simulink model "ofdm_txrx_mimo"  (Simulink 7.1)
settings: Solver=ode45  StartTime=0.0  StopTime=6e3  RelTol=1e-3
PreLoadFcn:
  | ofdm_rx_mimo_init
InitFcn:
  | ofdm_rx_mimo_init
StartFcn:
  | ofdm_rx_mimo_init
values from neighbouring files (symbols this model uses):
  BER_RAM_init_size = 2048    [ofdm_tx_mimo_init.m]
  CPLength = 16    [ofdm_tx_mimo_init.m]
  CRCPolynomial = hex2dec('04c11db7')    [ofdm_tx_mimo_init.m]
  freqOffset_track_filtCoef_i = hex2dec('2500')/2^32    [ofdm_rx_mimo_init.m]
  freqOffset_track_filtCoef_p = hex2dec('d0000')/2^32    [ofdm_rx_mimo_init.m]
  hi = zeros(1,length(cci))    [ofdm_rx_mimo_init.m]
  hr = zeros(1,length(ccr))    [ofdm_rx_mimo_init.m]
  max_CP_length = 16    [ofdm_tx_mimo_init.m]
  max_num_baseRateSymbols = 31    [ofdm_tx_mimo_init.m]
  max_num_subcarriers = 64    [ofdm_tx_mimo_init.m]
  max_num_trainingSymbols = 15    [ofdm_tx_mimo_init.m]
  max_numBytes = 16384    [ofdm_tx_mimo_init.m]
  max_OFDM_symbols = 2047    [ofdm_tx_mimo_init.m]
  modConstellation_qam16 = .75*[1 3 -1 -3]./3    [ofdm_tx_mimo_init.m]
  modConstellation_qam256 = 0.9375*[3 1 5 7 11 9 13 15 -3 -1 -5 -7 -11 -9 -13 -15]./15    [ofdm_tx_mimo_init.m]
  modConstellation_qam64 = 0.875*[3 1 5 7 -3 -1 -5 -7]./7    [ofdm_tx_mimo_init.m]
  modConstellation_qpsk = [1 -1]./sqrt(2)    [ofdm_tx_mimo_init.m]
  numBaseRateSymbols = 2    [ofdm_tx_mimo_init.m]
  numSubcarriers = 64    [ofdm_tx_mimo_init.m]
  numTrainingSymbols = 2    [ofdm_tx_mimo_init.m]
  pilot1_indicies = 7 + ( (64-7) * 2^16)    (= 3735559)    [ofdm_tx_mimo_init.m]
  pilot2_indicies = 21 + ( (64-21) * 2^16)    (= 2818069)    [ofdm_tx_mimo_init.m]
  pilotValue_neg = hex2dec('8000') + (2^16 * 0)    [ofdm_tx_mimo_init.m]
  pilotValue_pos = hex2dec('7FFF') + (2^16 * 0)    [ofdm_tx_mimo_init.m]
  pkt_crossCorr_thresh = 0.7    [ofdm_rx_mimo_init.m]
  pkt_energy_thresh = 0    [ofdm_rx_mimo_init.m]
  pkt_numPayloadBytes = 1400+42-6    (= 1436)    [ofdm_tx_mimo_init.m]
  pktByteNums = numHeaderBytes +  				byteIndex_numPayloadBytes(1)*2^8 +  				byteIndex_numPayloadBytes(2)*2^16 +  				byteIndex_simpleDynModMasks * 2^24    [ofdm_rx_mimo_init.m]
  PktDet_Delay = 26 + Symbol_Timing_Offset*2^7    (= 1050)    [ofdm_rx_mimo_init.m]
  preamble = 6*[0 shortsyms_10 longsyms_2]    [ofdm_tx_mimo_init.m]
  preamble_I = real(preamble)    [ofdm_tx_mimo_init.m]
  preamble_Q = imag(preamble)    [ofdm_tx_mimo_init.m]
  RAM_init_size = max_numBytes    (= 16384)    [ofdm_tx_mimo_init.m]
  reg_Interrupt_PktBuf_Control = reg_InterruptControl +  	1 * 2^16 +  	0 * 2^24    (= 65656)    [ofdm_rx_mimo_init.m]
  rx_controlBits = 1 * 2  		 + 1 * 4  		 + 0 * 8  		 + 1 * 16 * rx_SISO_Mode  		 + 1 * 32  		 + 1 * 64  		 + 0 * 128  		 + 1 * 256  		 + 0 * 512  		 + 1 * 1024  		 + 0 * 2048  		 + 1 * 4096  		 + 1 * 8192  		 + 0 * 16384  		 + 1 * 65536  		 + 1 * 131072  		 + 0 * 262144  		 + 1 * 524288    [ofdm_rx_mimo_init.m]
  Rx_PhaseNoiseTrack_K = 0.6094    [ofdm_rx_mimo_init.m]
  rx_postEq_scaling = round(rxScaling*2^11) * (1 + 2^16)    [ofdm_rx_mimo_init.m]
  RxDataScrambling_Seq = [40 198 78 63 82 173 102 245 48 111 172 115 147 230 216 93 72 65 62 2 205 242 122 90 128 83 105 97 73 10 5 252]    [ofdm_tx_mimo_init.m]
  simOnly_numSamples = length(preamble)+( (numSubcarriers+CPLength)*(numTrainingSymbols + numBaseRateSymbols + numFullRateSymbols/2) )    [ofdm_tx_mimo_init.m]
  train = [train train]    [ofdm_tx_mimo_init.m]
  tx_controlBits = (antB_preambleShift * 2^4) + (2^0 * tx_SISO_Mode) + 4*0 + 8*1    (= 73)    [ofdm_tx_mimo_init.m]
  TxDataScrambling_Seq = [40 198 78 63 82 173 102 245 48 111 172 115 147 230 216 93 72 65 62 2 205 242 122 90 128 83 105 97 73 10 5 252]    [ofdm_tx_mimo_init.m]
  txReg_symbolCounts = (2^16 * numFullRateSymbols) + (2^8 *numBaseRateSymbols ) + numTrainingSymbols    [ofdm_tx_mimo_init.m]
  TxRx_FFTScaling = bin2dec('010111') + (bin2dec('000101') * 2^6)    [ofdm_tx_mimo_init.m]
undefined symbols (not defined in the file or in its folder): AntA, AntB, BaseRate, CRC_table_gen, gcbh, intfilt, pktTiming_count_payloadStart, pktTiming_symbol_length, xlBlockLoadCallback, xlGetSimulinkPeriod
libraries: xbsIndex_r4 x4212, dspmlti4 x4, dspsigops x4, commchan3 x2, simulink x2, commrflib2 x2, dspsrcs4 x1
(part 11 of 43)

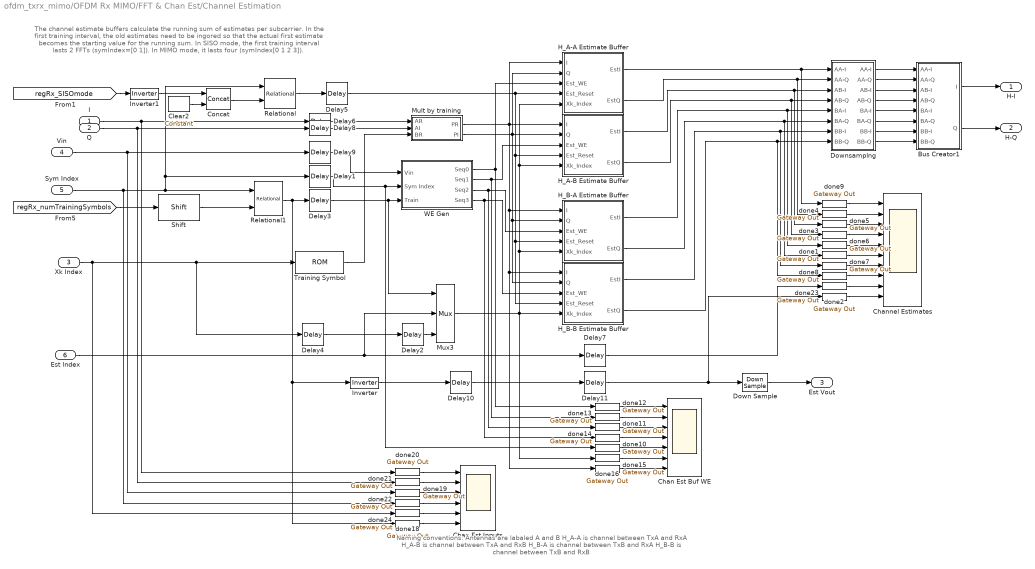
== system ofdm_txrx_mimo/OFDM Rx MIMO/FFT & Chan Est/Channel Estimation ==
  note: The channel estimate buffers calculate the
      | running sum of estimates per subcarrier. In the first
      | training interval, the old estimates need to be ingored
      | so that the actual first estimate becomes the starting value
      | for the running sum. In SISO mode, the first training
      | interval lasts 2 FFTs (symIndex=[0 1]). In MIMO
      | mode, it lasts four (symIndex[0 1 2 3]).
  note: Naming conventions:
      | Antennas are labaled A and B
      | H_A-A is channel between TxA and RxA
      | H_A-B is channel between TxA and RxB
      | H_B-A is channel between TxB and RxA
      | H_B-B is channel between TxB and RxB
  I : Inport  OutDataType=sfix(16)  OutScaling=2^0
  Q : Inport  Port=2  OutDataType=sfix(16)  OutScaling=2^0
  Xk Index : Inport  Port=3  OutDataType=sfix(16)  OutScaling=2^0
  Vin : Inport  Port=4  OutDataType=sfix(16)  OutScaling=2^0
  Sym Index : Inport  Port=5  OutDataType=sfix(16)  OutScaling=2^0
  Est Index : Inport  Port=6  OutDataType=sfix(16)  OutScaling=2^0
  Bus Creator1 : Subsystem
  Chan Est Buf WE : Scope  NumInputPorts=7
      settings: TimeRange=2000  YMin=0~0~0~0~0~0~-0.4  YMax=1~1~1~1~30~70~0.4  SaveName=ScopeData21
  Chan Est Inputs : Scope  NumInputPorts=6
      settings: TimeRange=1800  YMin=-0.01~-0.01~0~0~0~0  YMax=0.01~0.01~1~10~80~1  SaveName=ScopeData23
  Channel Estimates : Scope  NumInputPorts=10
      settings: TimeRange=3000  YMin=-0.2~-0.2~-1~-1~-1~-1~-1~-1~0~0.95  YMax=0.2~0.2~1~1~1~1~1~1~75~1.05  SaveName=ScopeData24
  Clear2 : xbsIndex_r4/Constant  arith_type=Unsigned  const=1  n_bits=1  bin_pt=0  explicit_period=off  period=1  equ=P=C  opselect=C  inp2=PCIN>>17  opr=+  inp1=P  carry=CIN  dbl_ovrd=off  block_type=constant
      settings: block_version=VER_STRING_GOES_HERE
  Concat : xbsIndex_r4/Concat  num_inputs=2  dbl_ovrd=off  block_type=concat
      settings: block_version=8.2.01
  Delay1 : xbsIndex_r4/Delay  en=off  latency=1  dbl_ovrd=off  reg_retiming=on  block_type=delay
      settings: infoedit=Hardware notes: A delay line is a chain, each link of which is an SRL16 followed by a flip-flop. If register retiming is enabled, the delay line is a chain of flip-flops.  xl_area=[0, 0, 0, 0, 0, 0, 0]  block_version=VER_STRING_GOES_HERE
  Delay{2,4..11} (9 alike) : xbsIndex_r4/Delay  en=off  latency=1  dbl_ovrd=off  reg_retiming=off  block_type=delay
      settings: infoedit=Hardware notes: A delay line is a chain, each link of which is an SRL16 followed by a flip-flop. If register retiming is enabled, the delay line is a chain of flip-flops.  xl_area=[0, 0, 0, 0, 0, 0, 0]  block_version=VER_STRING_GOES_HERE
  Delay3 : xbsIndex_r4/Delay  en=off  latency=1  dbl_ovrd=off  reg_retiming=on  block_type=delay
      settings: infoedit=Hardware notes: A delay line is a chain, each link of which is an SRL16 followed by a flip-flop. If register retiming is enabled, the delay line is a chain of flip-flops.  xl_area=[0, 0, 0, 0, 0, 0, 0]  block_version=VER_STRING_GOES_HERE
  Down Sample : xbsIndex_r4/Down Sample  sample_ratio=2  sample_phase="Last Value of Frame  (most efficient)"  en=off  latency=1  dbl_ovrd=off  block_type=dsamp
      settings: block_version=10.1.2
  Downsamplng : Subsystem
  H_A-A Estimate Buffer : Subsystem
  H_A-B Estimate Buffer : Subsystem
  H_B-A Estimate Buffer : Subsystem
  H_B-B Estimate Buffer : Subsystem
  Inverter : xbsIndex_r4/Inverter  en=off  latency=1  dbl_ovrd=off  block_type=inv
      settings: block_version=VER_STRING_GOES_HERE
  Inverter1 : xbsIndex_r4/Inverter  en=off  latency=0  dbl_ovrd=off  block_type=inv
      settings: block_version=VER_STRING_GOES_HERE
  Mult by training : Subsystem
  Mux3 : xbsIndex_r4/Mux  inputs=2  en=off  latency=0  precision=Full  arith_type="Signed  (2's comp)"  n_bits=8  bin_pt=2  quantization=Truncate  overflow=Wrap  dbl_ovrd=off  block_type=mux
      settings: xl_area=[0, 0, 0, 0, 0, 0, 0]  block_version=VER_STRING_GOES_HERE
  Relational : xbsIndex_r4/Relational  mode=a<=b  en=off  latency=0  dbl_ovrd=off  block_type=relational
      settings: xl_area=[0, 0, 0, 0, 0, 0, 0]  block_version=VER_STRING_GOES_HERE
  Relational1 : xbsIndex_r4/Relational  mode=a<b  en=off  latency=0  dbl_ovrd=off  block_type=relational
      settings: xl_area=[3 0 0 6 0 0 0]  block_version=VER_STRING_GOES_HERE
  Shift : xbsIndex_r4/Shift  shift_dir=Left  shift_bits=1  en=off  latency=0  precision=User Defined  arith_type=Unsigned  n_bits=1+ceil(log2(max_num_trainingSymbols))  bin_pt=0  quantization=Truncate  overflow=Flag as error  dbl_ovrd=off  block_type=shift
      settings: block_version=VER_STRING_GOES_HERE
  Training Symbol : xbsIndex_r4/ROM  depth=length(train)/2  initVector=sign(train)  distributed_mem=Block RAM  rst=off  init_reg=0  en=off  latency=1  arith_type="Signed  (2's comp)"  n_bits=2  bin_pt=1  dbl_ovrd=off  optimize=Area  use_rpm=off  block_type=sprom
      settings: xl_area=[0, 0, 0, 0, 0, 0, 0]  block_version=VER_STRING_GOES_HERE
  WE Gen : Subsystem
  done{1..16,18..24} (23 alike) : xbsIndex_r4/Gateway Out  hdl_port=off  timing_constraint=None  locs_specified=off  LOCs={}  block_type=gatewayout
      settings: infoedit="Gateway out block.  Converts Xilinx fixed point inputs into ouputs of type Simulink integer, double, or fixed point.<P><P>Hardware notes:  In hardware these blocks become top level output ports or are discarded, depending on how they are configured."  xl_area=[0 0 0 0 1 0 0]  block_version=VER_STRING_GOES_HERE
  H-I : Outport  OutDataType=sfix(16)  OutScaling=2^0
  H-Q : Outport  Port=2  OutDataType=sfix(16)  OutScaling=2^0
  Est Vout : Outport  Port=3  OutDataType=sfix(16)  OutScaling=2^0
  wires:
  Inverter1/1 -> Concat/1
  Clear2/1 -> Concat/2
  [regRx_SISOmode] -> Inverter1/1
  Delay5/1 -> H_B-B Estimate Buffer/4(Est_Reset), H_B-A Estimate Buffer/4(Est_Reset), H_A-B Estimate Buffer/4(Est_Reset), H_A-A Estimate Buffer/4(Est_Reset)
  Delay2/1 -> Mux3/3
  Est Index/1 -> Mux3/2, Delay7/1
  Delay10/1 -> Delay11/1
  done23/1 -> Channel Estimates/10
  done9/1 -> Channel Estimates/1
  done2/1 -> Channel Estimates/9
  done8/1 -> Channel Estimates/8
  done7/1 -> Channel Estimates/7
  done6/1 -> Channel Estimates/5
  done3/1 -> Channel Estimates/4
  done5/1 -> Channel Estimates/3
  done4/1 -> Channel Estimates/2
  done1/1 -> Channel Estimates/6
  H_B-B Estimate Buffer/2(EstQ) -> done8/1, Downsamplng/8(BB-Q)
  H_B-B Estimate Buffer/1(EstI) -> done7/1, Downsamplng/7(BB-I)
  H_B-A Estimate Buffer/2(EstQ) -> done1/1, Downsamplng/6(BA-Q)
  H_B-A Estimate Buffer/1(EstI) -> done6/1, Downsamplng/5(BA-I)
  H_A-B Estimate Buffer/2(EstQ) -> done3/1, Downsamplng/4(AB-Q)
  H_A-B Estimate Buffer/1(EstI) -> done5/1, Downsamplng/3(AB-I)
  H_A-A Estimate Buffer/2(EstQ) -> done4/1, Downsamplng/2(AA-Q)
  H_A-A Estimate Buffer/1(EstI) -> done9/1, Downsamplng/1(AA-I)
  Delay11/1 -> done23/1, Down Sample/1
  Inverter/1 -> Delay10/1
  Delay7/1 -> done2/1
  done24/1 -> Chan Est Inputs/6
  done22/1 -> Chan Est Inputs/4
  done19/1 -> Chan Est Inputs/3
  done21/1 -> Chan Est Inputs/2
  done20/1 -> Chan Est Inputs/1
  done18/1 -> Chan Est Inputs/5
  Delay9/1 -> WE Gen/1(Vin)
  Vin/1 -> done19/1, Delay9/1
  Delay8/1 -> Mult by training/2(AI)
  Delay6/1 -> Mult by training/1(AR)
  Delay3/1 -> Mux3/1, WE Gen/3(Train)
  Delay1/1 -> done10/1, WE Gen/2(Sym Index)
  [regRx_numTrainingSymbols] -> Shift/1
  done15/1 -> Chan Est Buf WE/7
  done16/1 -> Chan Est Buf WE/6
  done14/1 -> Chan Est Buf WE/4
  done11/1 -> Chan Est Buf WE/3
  done13/1 -> Chan Est Buf WE/2
  done12/1 -> Chan Est Buf WE/1
  done10/1 -> Chan Est Buf WE/5
  Mult by training/2(PI) -> H_B-B Estimate Buffer/2(Q), H_B-A Estimate Buffer/2(Q), H_A-A Estimate Buffer/2(Q), H_A-B Estimate Buffer/2(Q)
  Relational1/1 -> Inverter/1, done24/1, Delay3/1
  WE Gen/2(Seq1) -> done13/1, H_A-B Estimate Buffer/3(Est_WE)
  WE Gen/4(Seq3) -> done14/1, H_B-B Estimate Buffer/3(Est_WE)
  WE Gen/3(Seq2) -> done11/1, H_B-A Estimate Buffer/3(Est_WE)
  WE Gen/1(Seq0) -> done12/1, H_A-A Estimate Buffer/3(Est_WE)
  Mux3/1 -> done16/1, H_A-A Estimate Buffer/5(Xk_Index), H_A-B Estimate Buffer/5(Xk_Index), H_B-A Estimate Buffer/5(Xk_Index), H_B-B Estimate Buffer/5(Xk_Index)
  Mult by training/1(PR) -> done15/1, H_B-B Estimate Buffer/1(I), H_B-A Estimate Buffer/1(I), H_A-B Estimate Buffer/1(I), H_A-A Estimate Buffer/1(I)
  Relational/1 -> Delay5/1
  Concat/1 -> Relational/2
  Xk Index/1 -> done18/1, Training Symbol/1, Delay4/1
  Sym Index/1 -> done22/1, Relational/1, Delay1/1, Relational1/1
  Q/1 -> done21/1, Delay8/1
  I/1 -> done20/1, Delay6/1
  Shift/1 -> Relational1/2
  Delay4/1 -> Delay2/1
  Training Symbol/1 -> Mult by training/3(BR)
  Down Sample/1 -> Est Vout/1
  Bus Creator1/1(I) -> H-I/1
  Bus Creator1/2(Q) -> H-Q/1
  Downsamplng/1(" AA-I") -> Bus Creator1/1(AA-I)
  Downsamplng/2(" AA-Q") -> Bus Creator1/2(AA-Q)
  Downsamplng/3(" AB-I") -> Bus Creator1/3(AB-I)
  Downsamplng/4(" AB-Q") -> Bus Creator1/4(AB-Q)
  Downsamplng/5(" BA-I") -> Bus Creator1/5(BA-I)
  Downsamplng/6(" BA-Q") -> Bus Creator1/6(BA-Q)
  Downsamplng/7(" BB-I") -> Bus Creator1/7(BB-I)
  Downsamplng/8(" BB-Q") -> Bus Creator1/8(BB-Q)

== system ofdm_txrx_mimo/OFDM Rx MIMO/FFT & Chan Est/FFT ==
  xn_I : Inport  OutDataType=sfix(16)  OutScaling=2^0
  xn_Q : Inport  Port=2  OutDataType=sfix(16)  OutScaling=2^0
  Start : Inport  Port=3  OutDataType=sfix(16)  OutScaling=2^0
  6-Bit Reverser : xbsIndex_r4/BitBasher  bitexpr=|  display_expr=off  sr_1=1  arith_type1=Unsigned  bin_pt1=0  sr_2=2  arith_type2=Unsigned  bin_pt2=0  sr_3=3  arith_type3=Unsigned  bin_pt3=0  sr_4=4  arith_type4=Unsigned  bin_pt4=0  dbl_ovrd=off  block_type=bitbasher
      | <html><head><meta name="qrichtext" content="1" /><style type="text/css">
      | p, li { white-space: pre-wrap; }
      | </style></head><body style=" font-family:'MS Shell Dlg 2'; font-size:8.25pt; font-weight:400; font-style:normal;">
      | <p style=" margin-top:12px; margin-bottom:12px; margin-left:0px; margin-right:0px; -qt-block-indent:0; text-indent:0px; font-family:'MS Shell Dlg'; font-size:8pt;">q={a[0], a[1], a[2], a[3], a[4], a[5]}</p></body></html>
      settings: block_version=10.1.2
  Constant3 : xbsIndex_r4/Constant  arith_type=Boolean  const=1  n_bits=1  bin_pt=0  explicit_period=off  period=1  equ=P=C  opselect=C  inp2=PCIN>>17  opr=+  inp1=P  carry=CIN  dbl_ovrd=off  block_type=constant
      settings: block_version=VER_STRING_GOES_HERE
  Constant4 : xbsIndex_r4/Constant  arith_type=Boolean  const=1  n_bits=1  bin_pt=0  explicit_period=off  period=1  equ=P=C  opselect=C  inp2=PCIN>>17  opr=+  inp1=P  carry=CIN  dbl_ovrd=off  block_type=constant
      settings: block_version=VER_STRING_GOES_HERE
  Constant6 : xbsIndex_r4/Constant  arith_type=Boolean  const=1  n_bits=1  bin_pt=0  explicit_period=off  period=1  equ=P=C  opselect=C  inp2=PCIN>>17  opr=+  inp1=P  carry=CIN  dbl_ovrd=off  block_type=constant
      settings: block_version=VER_STRING_GOES_HERE
  Convert : xbsIndex_r4/Convert  arith_type="Signed  (2's comp)"  n_bits=16  bin_pt=15  quantization=Truncate  overflow=Flag as error  latency=0  dbl_ovrd=off  pipeline=off  block_type=convert
      settings: block_version=VER_STRING_GOES_HERE
  Convert1 : xbsIndex_r4/Convert  arith_type="Signed  (2's comp)"  n_bits=16  bin_pt=15  quantization=Truncate  overflow=Flag as error  latency=0  dbl_ovrd=off  pipeline=off  block_type=convert
      settings: block_version=VER_STRING_GOES_HERE
  FFT IO : Scope  NumInputPorts=6
      settings: TimeRange=4000  YMin=-1~-1~0~0~0~-1  YMax=1~1~65~1~1~1  SaveName=ScopeData61
  FFT Input : Scope  NumInputPorts=4
      settings: TimeRange=4000  YMin=-0.1~-0.2~0~0  YMax=0.15~0.8~1~80  SaveName=ScopeData14
  FFT Output : Scope  NumInputPorts=6
      settings: TimeRange=4000  YMin=-1~-1~0~0~0~-1  YMax=1~1~65~1~1~1  SaveName=ScopeData15
  FFTx : xbsIndex_r4/FFT v3_1   arch=Pipelined Streaming I/O  npts=64  scaling=Scaled  rounding=Convergent Rounding  tf_width=16  en_n=off  has_ovflo=on  rst=on  en=off  op_order=Natural Order  dbl_ovrd=off  explicit_period=off  period=1  use_dsp48=off  twiddle_mem_type=Block RAM  data_mem_type=Block RAM  num_bram_stages=3  block_type=fftx
      settings: infoedit=Devices Supported: Virtex-4, Virtex-II, Virtex-IIPro, Spartan-3 and Spartan-3E  xl_area=[0, 0, 0, 0, 0, 0, 0]  block_version=VER_STRING_GOES_HERE
  Up Sample1 : xbsIndex_r4/Up Sample  sample_ratio=2  copy_samples=on  dbl_ovrd=off  block_type=usamp
      settings: infoedit="Up samples input data.  Inserted values  can be zeros or copies of the most recent input sample.<P><P>Hardware notes: No hardware is needed if inserted values are copies of the input sample; otherwise, a mux and single bit flip-flop are used."  block_version=VER_STRING_GOES_HERE
  Up Sample2 : xbsIndex_r4/Up Sample  sample_ratio=2  copy_samples=on  dbl_ovrd=off  block_type=usamp
      settings: infoedit="Up samples input data.  Inserted values  can be zeros or copies of the most recent input sample.<P><P>Hardware notes: No hardware is needed if inserted values are copies of the input sample; otherwise, a mux and single bit flip-flop are used."  block_version=VER_STRING_GOES_HERE
  done{1..10} (10 alike) : xbsIndex_r4/Gateway Out  hdl_port=off  timing_constraint=None  locs_specified=off  LOCs={}  block_type=gatewayout
      settings: infoedit="Gateway out block.  Converts Xilinx fixed point inputs into ouputs of type Simulink integer, double, or fixed point.<P><P>Hardware notes:  In hardware these blocks become top level output ports or are discarded, depending on how they are configured."  xl_area=[0 0 0 0 1 0 0]  block_version=VER_STRING_GOES_HERE
  xk_I : Outport  OutDataType=sfix(16)  OutScaling=2^0
  xk_Q : Outport  Port=2  OutDataType=sfix(16)  OutScaling=2^0
  xk_Index : Outport  Port=3  OutDataType=sfix(16)  OutScaling=2^0
  Vout : Outport  Port=4  OutDataType=sfix(16)  OutScaling=2^0
  Done : Outport  Port=5  OutDataType=sfix(16)  OutScaling=2^0
  xn_Index : Outport  Port=6  OutDataType=sfix(16)  OutScaling=2^0
  wires:
  6-Bit Reverser/1 -> FFT IO/6
  [Rx_FFTScaling] -> Up Sample2/1
  Constant6/1 -> FFTx/7
  FFTx/10 -> done1/1
  done1/1 -> FFT Output/6
  FFTx/9 -> FFT IO/4, Done/1, done2/1
  done2/1 -> FFT Output/5
  done10/1 -> FFT Output/4
  done7/1 -> FFT Output/3
  done9/1 -> FFT Output/2
  done8/1 -> FFT Output/1
  done6/1 -> FFT Input/4
  done3/1 -> FFT Input/3
  done5/1 -> FFT Input/2
  done4/1 -> FFT Input/1
  Convert1/1 -> done5/1, FFTx/2
  Convert/1 -> done4/1, FFTx/1
  FFTx/7 -> FFT IO/5, done10/1, Vout/1
  FFTx/4 -> 6-Bit Reverser/1, FFT IO/3, done7/1, xk_Index/1
  Start/1 -> FFT IO/1, done3/1, FFTx/3
  FFTx/3 -> FFT IO/2, done6/1, xn_Index/1
  xn_Q/1 -> Convert1/1
  FFTx/2 -> done9/1, xk_Q/1
  FFTx/1 -> done8/1, xk_I/1
  xn_I/1 -> Convert/1
  [rx_global_reset] -> Up Sample1/1
  Constant4/1 -> FFTx/5
  Constant3/1 -> FFTx/4
  Up Sample1/1 -> FFTx/8
  Up Sample2/1 -> FFTx/6

== system ofdm_txrx_mimo/OFDM Rx MIMO/FFT & Chan Est/Input Buffers ==
  AntA I : Inport  OutDataType=sfix(16)  OutScaling=2^0
  AntA Q : Inport  Port=2  OutDataType=sfix(16)  OutScaling=2^0
  AntB I : Inport  Port=3  OutDataType=sfix(16)  OutScaling=2^0
  AntB Q : Inport  Port=4  OutDataType=sfix(16)  OutScaling=2^0
  We : Inport  Port=5  OutDataType=sfix(16)  OutScaling=2^0
  Wr Addr : Inport  Port=6  OutDataType=sfix(16)  OutScaling=2^0
  Rd Addr : Inport  Port=7  OutDataType=sfix(16)  OutScaling=2^0
  Ant Sel : Inport  Port=8  OutDataType=sfix(16)  OutScaling=2^0
  Antenna A RAM : Subsystem
  Antenna B RAM : Subsystem
  Mux : xbsIndex_r4/Mux  inputs=2  en=off  latency=1  precision=Full  arith_type=Unsigned  n_bits=16  bin_pt=14  quantization=Truncate  overflow=Wrap  dbl_ovrd=off  block_type=mux
      settings: block_version=VER_STRING_GOES_HERE
  Mux1 : xbsIndex_r4/Mux  inputs=2  en=off  latency=1  precision=Full  arith_type=Unsigned  n_bits=16  bin_pt=14  quantization=Truncate  overflow=Wrap  dbl_ovrd=off  block_type=mux
      settings: block_version=VER_STRING_GOES_HERE
  Register : xbsIndex_r4/Register  init=0  rst=off  en=off  dbl_ovrd=off  block_type=register
      settings: block_version=VER_STRING_GOES_HERE
  Register1 : xbsIndex_r4/Register  init=0  rst=off  en=off  dbl_ovrd=off  block_type=register
      settings: block_version=VER_STRING_GOES_HERE
  Rx Buffers : Scope  NumInputPorts=6
      settings: TimeRange=2500  YMin=-0.015~-0.015~0~0~0~-5  YMax=0.015~0.015~80~80~1~5  SaveName=ScopeData19
  Rx Buffers Out : Scope  NumInputPorts=7
      settings: TimeRange=2500  YMin=-0.2~-0.7~0~-0.2~-0.7~-0.2~0  YMax=0.5~0.3~0.5~0.5~0~0.25~1  SaveName=ScopeData32
  done{1..13} (13 alike) : xbsIndex_r4/Gateway Out  hdl_port=off  timing_constraint=None  locs_specified=off  LOCs={}  block_type=gatewayout
      settings: infoedit="Gateway out block.  Converts Xilinx fixed point inputs into ouputs of type Simulink integer, double, or fixed point.<P><P>Hardware notes:  In hardware these blocks become top level output ports or are discarded, depending on how they are configured."  xl_area=[0 0 0 0 1 0 0]  block_version=VER_STRING_GOES_HERE
  " I" : Outport  OutDataType=sfix(16)  OutScaling=2^0
  " Q" : Outport  Port=2  OutDataType=sfix(16)  OutScaling=2^0
  wires:
  done13/1 -> Rx Buffers Out/7
  done3/1 -> Rx Buffers Out/6
  done5/1 -> Rx Buffers Out/5
  done4/1 -> Rx Buffers Out/4
  done6/1 -> Rx Buffers Out/3
  done12/1 -> Rx Buffers Out/2
  done11/1 -> Rx Buffers Out/1
  done1/1 -> Rx Buffers/6  "AntA I Out"
  done2/1 -> Rx Buffers/5  "Write En"
  done10/1 -> Rx Buffers/4  "Rd Addr"
  done7/1 -> Rx Buffers/3  "Write Addr"
  done9/1 -> Rx Buffers/2  "AntQ Q"
  done8/1 -> Rx Buffers/1  "AntA I"
  Register1/1 -> done13/1, " Q"/1
  Register/1 -> done3/1, " I"/1
  Mux1/1 -> Register1/1
  Mux/1 -> Register/1
  Ant Sel/1 -> done11/1, Mux1/1, Mux/1
  Antenna B RAM/1(" I") -> done4/1, Mux/3
  Antenna B RAM/2(" Q") -> done5/1, Mux1/3
  Antenna A RAM/2(" Q") -> done6/1, Mux1/2
  Antenna A RAM/1(" I") -> done1/1, done12/1, Mux/2
  Rd Addr/1 -> Antenna B RAM/5(Rd Addr), done10/1, Antenna A RAM/5(Rd Addr)
  We/1 -> done2/1, Antenna B RAM/3(We), Antenna A RAM/3(We)
  Wr Addr/1 -> Antenna B RAM/4(Wr Addr), done7/1, Antenna A RAM/4(Wr Addr)
  AntB Q/1 -> Antenna B RAM/2(Q)
  AntB I/1 -> Antenna B RAM/1(I)
  AntA Q/1 -> done9/1, Antenna A RAM/2(Q)
  AntA I/1 -> done8/1, Antenna A RAM/1(I)

== system ofdm_txrx_mimo/OFDM Rx MIMO/FFT & Chan Est/Output Buffers ==
  I : Inport  OutDataType=sfix(16)  OutScaling=2^0
  Q : Inport  Port=2  OutDataType=sfix(16)  OutScaling=2^0
  Wr Addr : Inport  Port=3  OutDataType=sfix(16)  OutScaling=2^0
  FFT Vout : Inport  Port=4  OutDataType=sfix(16)  OutScaling=2^0
  SymIndex : Inport  Port=5  OutDataType=sfix(16)  OutScaling=2^0
  Rd Addr : Inport  Port=6  OutDataType=sfix(16)  OutScaling=2^0
  AntA Buffer : Subsystem
  AntB Buffer : Subsystem
  Bus Creator1 : BusCreator  Inputs=2
  Bus Creator2 : BusCreator  Inputs=2
  FFT Output Buffers : Scope  NumInputPorts=10
      settings: TimeRange=4000  YMin=-0.4~-0.4~0~0~0~0~-0.4~-0.4~0~0  YMax=0.4~0.4~70~1~1~70~0.4~0.8~0.0125~0.8  SaveName=ScopeData53
  Inverter : xbsIndex_r4/Inverter  en=off  latency=0  dbl_ovrd=off  block_type=inv
      settings: block_version=VER_STRING_GOES_HERE
  LSB : xbsIndex_r4/Slice  nbits=1  boolean_output=on  mode=Upper Bit Location + Width  bit1=0  base1=LSB of Input  bit0=0  base0=LSB of Input  dbl_ovrd=off  block_type=slice
      settings: infoedit="Extracts a given range of bits from each input sample and presents it at the output.  The output type is ordinarily unsigned with binary point at zero, but can be Boolean when the slice is one bit wide.<P><P>Hardware notes: In hardware this block costs nothing."  block_version=VER_STRING_GOES_HERE
  Logical1 : xbsIndex_r4/Logical  logical_function=AND  inputs=2  en=off  latency=0  precision=Full  arith_type=Unsigned  n_bits=8  bin_pt=2  align_bp=on  dbl_ovrd=off  block_type=logical
      settings: xl_area=[0, 0, 0, 0, 0, 0, 0]  block_version=VER_STRING_GOES_HERE
  Logical2 : xbsIndex_r4/Logical  logical_function=AND  inputs=2  en=off  latency=0  precision=Full  arith_type=Unsigned  n_bits=8  bin_pt=2  align_bp=on  dbl_ovrd=off  block_type=logical
      settings: xl_area=[0, 0, 0, 0, 0, 0, 0]  block_version=VER_STRING_GOES_HERE
  done{1..10} (10 alike) : xbsIndex_r4/Gateway Out  hdl_port=off  timing_constraint=None  locs_specified=off  LOCs={}  block_type=gatewayout
      settings: infoedit="Gateway out block.  Converts Xilinx fixed point inputs into ouputs of type Simulink integer, double, or fixed point.<P><P>Hardware notes:  In hardware these blocks become top level output ports or are discarded, depending on how they are configured."  xl_area=[0 0 0 0 1 0 0]  block_version=VER_STRING_GOES_HERE
  " I" : Outport  OutDataType=sfix(16)  OutScaling=2^0
  " Q" : Outport  Port=2  OutDataType=sfix(16)  OutScaling=2^0
  wires:
  Bus Creator2/1 -> " Q"/1
  Bus Creator1/1 -> " I"/1
  done6/1 -> FFT Output Buffers/8  "A_Q"
  done5/1 -> FFT Output Buffers/7  "A_I"
  done4/1 -> FFT Output Buffers/10  "B_Q"
  done3/1 -> FFT Output Buffers/9  "B_I"
  done1/1 -> FFT Output Buffers/6  "Rd Addr"
  done2/1 -> FFT Output Buffers/4  "A WE"
  done10/1 -> FFT Output Buffers/5  "B WE"
  done7/1 -> FFT Output Buffers/3  "Wr Addr"
  done9/1 -> FFT Output Buffers/2  "Q"
  done8/1 -> FFT Output Buffers/1  "I"
  Inverter/1 -> Logical2/1
  Q/1 -> done9/1, AntA Buffer/2(Q), AntB Buffer/2(Q)
  I/1 -> done8/1, AntA Buffer/1(I), AntB Buffer/1(I)
  Wr Addr/1 -> done7/1, AntB Buffer/4(Wr Addr), AntA Buffer/4(Wr Addr)
  FFT Vout/1 -> Logical1/2, Logical2/2
  AntA Buffer/2(" Q") -> done6/1, Bus Creator2/2
  AntA Buffer/1(" I") -> done5/1, Bus Creator1/2
  AntB Buffer/2(" Q") -> done4/1, Bus Creator2/1
  AntB Buffer/1(" I") -> done3/1, Bus Creator1/1
  LSB/1 -> Inverter/1, Logical1/1
  Logical1/1 -> done10/1, AntB Buffer/3(We)
  Logical2/1 -> done2/1, AntA Buffer/3(We)
  SymIndex/1 -> LSB/1
  Rd Addr/1 -> done1/1, AntB Buffer/5(Rd Addr), AntA Buffer/5(Rd Addr)

== system ofdm_txrx_mimo/OFDM Rx MIMO/FFT & Chan Est/Symbol Counter ==
  note: SR Latch enables counter after the first symbol. This keeps
      | the symbol index at zero after the first FFT finishes so that
      | the symbol index, like xn and xk_index, is zero-indexed.
  FFT Done : Inport  OutDataType=sfix(16)  OutScaling=2^0
  Payload : Inport  Port=2  OutDataType=sfix(16)  OutScaling=2^0
  Counter : xbsIndex_r4/Counter  cnt_type=Free Running  cnt_to=Inf  operation=Up  start_count=0  cnt_by_val=1  arith_type=Unsigned  n_bits=ceil(log2(max_OFDM_symbols+1))  bin_pt=0  load_pin=off  rst=on  en=on  explicit_period=off  period=1  dbl_ovrd=off  use_behavioral_HDL=off  use_rpm=on  implementation=Fabric  block_type=counter
      settings: xl_area=[6 12 0 12 0 0 0]  block_version=VER_STRING_GOES_HERE
  Inverter : xbsIndex_r4/Inverter  en=off  latency=0  dbl_ovrd=off  block_type=inv
      settings: xl_area=[0 0 0 0 0 0 0]  block_version=VER_STRING_GOES_HERE
  Logical1 : xbsIndex_r4/Logical  logical_function=AND  inputs=2  en=off  latency=0  precision=Full  arith_type=Unsigned  n_bits=8  bin_pt=2  align_bp=on  dbl_ovrd=off  block_type=logical
      settings: xl_area=[0, 0, 0, 0, 0, 0, 0]  block_version=VER_STRING_GOES_HERE
  Logical2 : xbsIndex_r4/Logical  logical_function=OR  inputs=2  en=off  latency=0  precision=Full  arith_type=Unsigned  n_bits=8  bin_pt=2  align_bp=on  dbl_ovrd=off  block_type=logical
      settings: xl_area=[4 0 0 4 0 0 0]  block_version=VER_STRING_GOES_HERE
  S-R Latch : Subsystem
  Up Sample : xbsIndex_r4/Up Sample  sample_ratio=4  copy_samples=on  dbl_ovrd=off  block_type=usamp
      settings: infoedit="Up samples input data.  Inserted values  can be zeros or copies of the most recent input sample.<P><P>Hardware notes: No hardware is needed if inserted values are copies of the input sample; otherwise, a mux and single bit flip-flop are used."  block_version=10.1.2
  Up Sample1 : xbsIndex_r4/Up Sample  sample_ratio=2  copy_samples=on  dbl_ovrd=off  block_type=usamp
      settings: infoedit="Up samples input data.  Inserted values  can be zeros or copies of the most recent input sample.<P><P>Hardware notes: No hardware is needed if inserted values are copies of the input sample; otherwise, a mux and single bit flip-flop are used."  block_version=10.1.2
  Sym Index : Outport  OutDataType=sfix(16)  OutScaling=2^0
  wires:
  Payload/1 -> Up Sample/1
  [rx_global_reset] -> Up Sample1/1
  Inverter/1 -> Logical2/1
  Logical2/1 -> S-R Latch/2(R), Counter/1
  S-R Latch/1(D) -> Logical1/2
  Logical1/1 -> Counter/2
  Counter/1 -> Sym Index/1
  FFT Done/1 -> S-R Latch/1(S), Logical1/1
  Up Sample/1 -> Inverter/1
  Up Sample1/1 -> Logical2/2

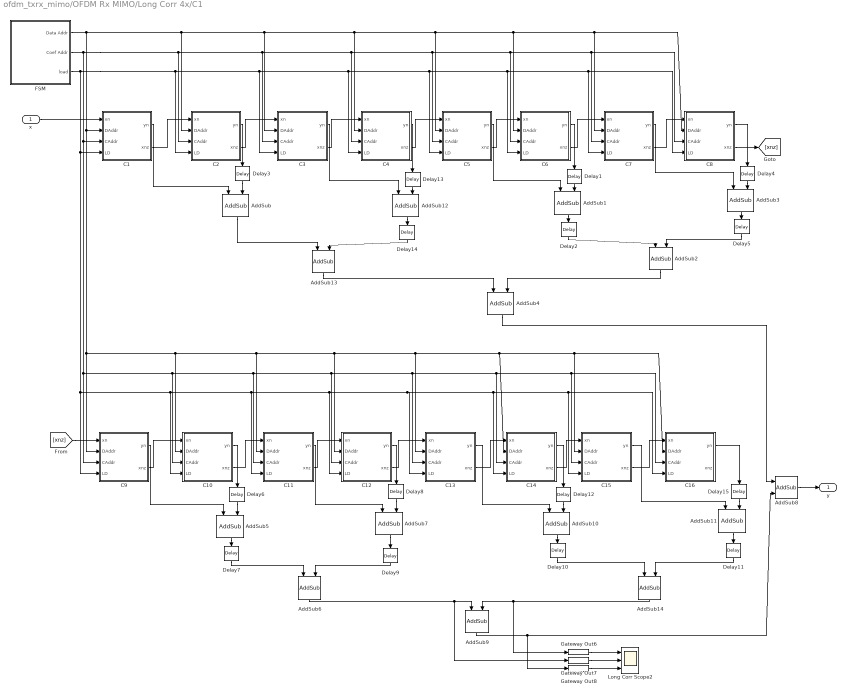
== system ofdm_txrx_mimo/OFDM Rx MIMO/Long Corr 4x/C1 ==
  x : Inport  OutDataType=sfix(16)  OutScaling=2^0
  AddSub : xbsIndex_r4/AddSub  Orientation=down  mode=Addition  use_carryin=off  use_carryout=off  en=off  latency=0  precision=Full  arith_type=Unsigned  n_bits=8  bin_pt=2  quantization=Truncate  overflow=Wrap  dbl_ovrd=off  use_behavioral_HDL=on  pipelined=off  use_rpm=off  hw_selection=Fabric  block_type=addsub
      settings: xl_area=[3 0 0 4 0 0 0]  block_version=VER_STRING_GOES_HERE
  AddSub{1..7,9..14} (13 alike) : xbsIndex_r4/AddSub  Orientation=down  mode=Addition  use_carryin=off  use_carryout=off  en=off  latency=1  precision=Full  arith_type=Unsigned  n_bits=8  bin_pt=2  quantization=Truncate  overflow=Wrap  dbl_ovrd=off  use_behavioral_HDL=on  pipelined=off  use_rpm=off  hw_selection=Fabric  block_type=addsub
      AddSub1 settings: xl_area=[3 5 0 4 0 0 0]  block_version=VER_STRING_GOES_HERE
      AddSub10 settings: xl_area=[3 5 0 4 0 0 0]  block_version=VER_STRING_GOES_HERE
      AddSub11 settings: xl_area=[3 5 0 4 0 0 0]  block_version=VER_STRING_GOES_HERE
      AddSub12 settings: xl_area=[3 5 0 4 0 0 0]  block_version=VER_STRING_GOES_HERE
      AddSub13 settings: xl_area=[3 6 0 5 0 0 0]  block_version=VER_STRING_GOES_HERE
      AddSub14 settings: xl_area=[3 6 0 5 0 0 0]  block_version=VER_STRING_GOES_HERE
      AddSub2 settings: xl_area=[3 6 0 5 0 0 0]  block_version=VER_STRING_GOES_HERE
      AddSub3 settings: xl_area=[3 5 0 4 0 0 0]  block_version=VER_STRING_GOES_HERE
      AddSub4 settings: xl_area=[4 7 0 6 0 0 0]  block_version=VER_STRING_GOES_HERE
      AddSub5 settings: xl_area=[3 5 0 4 0 0 0]  block_version=VER_STRING_GOES_HERE
      AddSub6 settings: xl_area=[3 6 0 5 0 0 0]  block_version=VER_STRING_GOES_HERE
      AddSub7 settings: xl_area=[3 5 0 4 0 0 0]  block_version=VER_STRING_GOES_HERE
      AddSub9 settings: xl_area=[4 7 0 6 0 0 0]  block_version=VER_STRING_GOES_HERE
  AddSub8 : xbsIndex_r4/AddSub  mode=Addition  use_carryin=off  use_carryout=off  en=off  latency=1  precision=Full  arith_type=Unsigned  n_bits=8  bin_pt=2  quantization=Truncate  overflow=Wrap  dbl_ovrd=off  use_behavioral_HDL=on  pipelined=off  use_rpm=off  hw_selection=Fabric  block_type=addsub
      settings: xl_area=[4 8 0 7 0 0 0]  block_version=VER_STRING_GOES_HERE
  C1 : Subsystem  mask{coef2=h(1:N)}
      mask prompts: coef2 "Coefficients"
  C10 : Subsystem  mask{coef2=h(9*N+1:10*N)}
      mask prompts: coef2 "Coefficients"
  C11 : Subsystem  mask{coef2=h(10*N+1:11*N)}
      mask prompts: coef2 "Coefficients"
  C12 : Subsystem  mask{coef2=h(11*N+1:12*N)}
      mask prompts: coef2 "Coefficients"
  C13 : Subsystem  mask{coef2=h(12*N+1:13*N)}
      mask prompts: coef2 "Coefficients"
  C14 : Subsystem  mask{coef2=h(13*N+1:14*N)}
      mask prompts: coef2 "Coefficients"
  C15 : Subsystem  mask{coef2=h(14*N+1:15*N)}
      mask prompts: coef2 "Coefficients"
  C16 : Subsystem  mask{coef2=h(15*N+1:16*N)}
      mask prompts: coef2 "Coefficients"
  C2 : Subsystem  mask{coef2=h(N+1:2*N)}
      mask prompts: coef2 "Coefficients"
  C3 : Subsystem  mask{coef2=h(2*N+1:3*N)}
      mask prompts: coef2 "Coefficients"
  C4 : Subsystem  mask{coef2=h(3*N+1:4*N)}
      mask prompts: coef2 "Coefficients"
  C5 : Subsystem  mask{coef2=h(4*N+1:5*N)}
      mask prompts: coef2 "Coefficients"
  C6 : Subsystem  mask{coef2=h(5*N+1:6*N)}
      mask prompts: coef2 "Coefficients"
  C7 : Subsystem  mask{coef2=h(6*N+1:7*N)}
      mask prompts: coef2 "Coefficients"
  C8 : Subsystem  mask{coef2=h(7*N+1:8*N)}
      mask prompts: coef2 "Coefficients"
  C9 : Subsystem  mask{coef2=h(8*N+1:9*N)}
      mask prompts: coef2 "Coefficients"
  Delay{1,3..4,6,8,12..13,15} (8 alike) : xbsIndex_r4/Delay  Orientation=down  en=off  latency=N-1  dbl_ovrd=off  reg_retiming=off  block_type=delay
      settings: infoedit=Hardware notes: A delay line is a chain, each link of which is an SRL16 followed by a flip-flop. If register retiming is enabled, the delay line is a chain of flip-flops.  xl_area=[2 4 0 4 0 0 0]  block_version=VER_STRING_GOES_HERE
  Delay10 : xbsIndex_r4/Delay  Orientation=down  en=off  latency=((N-1)*12)-1  dbl_ovrd=off  reg_retiming=off  block_type=delay
      settings: infoedit=Hardware notes: A delay line is a chain, each link of which is an SRL16 followed by a flip-flop. If register retiming is enabled, the delay line is a chain of flip-flops.  xl_area=[9 15 0 15 0 0 0]  block_version=VER_STRING_GOES_HERE
  Delay11 : xbsIndex_r4/Delay  Orientation=down  en=off  latency=((N-1)*14)-1  dbl_ovrd=off  reg_retiming=off  block_type=delay
      settings: infoedit=Hardware notes: A delay line is a chain, each link of which is an SRL16 followed by a flip-flop. If register retiming is enabled, the delay line is a chain of flip-flops.  xl_area=[9 15 0 15 0 0 0]  block_version=VER_STRING_GOES_HERE
  Delay14 : xbsIndex_r4/Delay  Orientation=down  en=off  latency=((N-1)*2)-1  dbl_ovrd=off  reg_retiming=off  block_type=delay
      settings: infoedit=Hardware notes: A delay line is a chain, each link of which is an SRL16 followed by a flip-flop. If register retiming is enabled, the delay line is a chain of flip-flops.  xl_area=[3 5 0 5 0 0 0]  block_version=VER_STRING_GOES_HERE
  Delay2 : xbsIndex_r4/Delay  Orientation=down  en=off  latency=((N-1)*4)-1  dbl_ovrd=off  reg_retiming=off  block_type=delay
      settings: infoedit=Hardware notes: A delay line is a chain, each link of which is an SRL16 followed by a flip-flop. If register retiming is enabled, the delay line is a chain of flip-flops.  xl_area=[3 5 0 5 0 0 0]  block_version=VER_STRING_GOES_HERE
  Delay5 : xbsIndex_r4/Delay  Orientation=down  en=off  latency=((N-1)*6)-1  dbl_ovrd=off  reg_retiming=off  block_type=delay
      settings: infoedit=Hardware notes: A delay line is a chain, each link of which is an SRL16 followed by a flip-flop. If register retiming is enabled, the delay line is a chain of flip-flops.  xl_area=[6 10 0 10 0 0 0]  block_version=VER_STRING_GOES_HERE
  Delay7 : xbsIndex_r4/Delay  Orientation=down  en=off  latency=((N-1)*8)-1  dbl_ovrd=off  reg_retiming=off  block_type=delay
      settings: infoedit=Hardware notes: A delay line is a chain, each link of which is an SRL16 followed by a flip-flop. If register retiming is enabled, the delay line is a chain of flip-flops.  xl_area=[6 10 0 10 0 0 0]  block_version=VER_STRING_GOES_HERE
  Delay9 : xbsIndex_r4/Delay  Orientation=down  en=off  latency=((N-1)*10)-1  dbl_ovrd=off  reg_retiming=off  block_type=delay
      settings: infoedit=Hardware notes: A delay line is a chain, each link of which is an SRL16 followed by a flip-flop. If register retiming is enabled, the delay line is a chain of flip-flops.  xl_area=[9 15 0 15 0 0 0]  block_version=VER_STRING_GOES_HERE
  FSM : Subsystem
  Gateway Out6 : xbsIndex_r4/Gateway Out  hdl_port=off  timing_constraint=None  locs_specified=off  LOCs={}  block_type=gatewayout
      settings: infoedit="Gateway out block.  Converts Xilinx fixed point inputs into ouputs of type Simulink integer, double, or fixed point.<P><P>Hardware notes:  In hardware these blocks become top level output ports or are discarded, depending on how they are configured."  xl_area=[0 0 0 0 0 0 0]  block_version=VER_STRING_GOES_HERE
  Gateway Out7 : xbsIndex_r4/Gateway Out  hdl_port=off  timing_constraint=None  locs_specified=off  LOCs={}  block_type=gatewayout
      settings: infoedit="Gateway out block.  Converts Xilinx fixed point inputs into ouputs of type Simulink integer, double, or fixed point.<P><P>Hardware notes:  In hardware these blocks become top level output ports or are discarded, depending on how they are configured."  xl_area=[0 0 0 0 0 0 0]  block_version=VER_STRING_GOES_HERE
  Gateway Out8 : xbsIndex_r4/Gateway Out  hdl_port=off  timing_constraint=None  locs_specified=off  LOCs={}  block_type=gatewayout
      settings: infoedit="Gateway out block.  Converts Xilinx fixed point inputs into ouputs of type Simulink integer, double, or fixed point.<P><P>Hardware notes:  In hardware these blocks become top level output ports or are discarded, depending on how they are configured."  xl_area=[0 0 0 0 0 0 0]  block_version=VER_STRING_GOES_HERE
  Long Corr Scope2 : Scope  NumInputPorts=3
      settings: YMin=-0.35~-0.175~0  YMax=0.35~0.225~1300  SaveName=ScopeData8
  y : Outport  OutDataType=sfix(16)  OutScaling=2^0
  wires:
  Gateway Out8/1 -> Long Corr Scope2/3
  Gateway Out7/1 -> Long Corr Scope2/2
  Gateway Out6/1 -> Long Corr Scope2/1
  AddSub14/1 -> Gateway Out6/1, AddSub9/2
  Delay11/1 -> AddSub14/2
  Delay10/1 -> AddSub14/1
  AddSub11/1 -> Delay11/1
  AddSub10/1 -> Delay10/1
  Delay15/1 -> AddSub11/2
  Delay12/1 -> AddSub10/2
  C16/1(yn) -> Delay15/1
  C14/1(yn) -> Delay12/1
  C15/1(yn) -> AddSub11/1
  C15/2(xnz) -> C16/1(xn)
  C14/2(xnz) -> C15/1(xn)
  C13/2(xnz) -> C14/1(xn)
  x/1 -> C1/1(xn)
  C2/1(yn) -> Delay3/1
  C1/2(xnz) -> C2/1(xn)
  FSM/1(Data Addr) -> C2/2(DAddr), C3/2(DAddr), C4/2(DAddr), C5/2(DAddr), C6/2(DAddr), C7/2(DAddr), C8/2(DAddr), C1/2(DAddr), C9/2(DAddr), C10/2(DAddr), C11/2(DAddr), C15/2(DAddr), C16/2(DAddr), C14/2(DAddr), C13/2(DAddr), C12/2(DAddr)
  FSM/2(Coef Addr) -> C2/3(CAddr), C3/3(CAddr), C4/3(CAddr), C5/3(CAddr), C6/3(CAddr), C7/3(CAddr), C8/3(CAddr), C1/3(CAddr), C9/3(CAddr), C10/3(CAddr), C11/3(CAddr), C15/3(CAddr), C16/3(CAddr), C14/3(CAddr), C13/3(CAddr), C12/3(CAddr)
  FSM/3(load) -> C2/4(LD), C3/4(LD), C4/4(LD), C5/4(LD), C6/4(LD), C7/4(LD), C8/4(LD), C1/4(LD), C9/4(LD), C10/4(LD), C11/4(LD), C15/4(LD), C16/4(LD), C14/4(LD), C13/4(LD), C12/4(LD)
  C2/2(xnz) -> C3/1(xn)
  C3/2(xnz) -> C4/1(xn)
  C3/1(yn) -> AddSub12/1
  C4/2(xnz) -> C5/1(xn)
  C4/1(yn) -> Delay13/1
  C5/2(xnz) -> C6/1(xn)
  C6/2(xnz) -> C7/1(xn)
  [xnz] -> C9/1(xn)
  C9/2(xnz) -> C10/1(xn)
  C10/2(xnz) -> C11/1(xn)
  C11/2(xnz) -> C12/1(xn)
  C12/2(xnz) -> C13/1(xn)
  Delay13/1 -> AddSub12/2
  AddSub12/1 -> Delay14/1
  Delay3/1 -> AddSub/2
  C1/1(yn) -> AddSub/1
  C5/1(yn) -> AddSub1/1
  Delay1/1 -> AddSub1/2
  AddSub1/1 -> Delay2/1
  C6/1(yn) -> Delay1/1
  C7/2(xnz) -> C8/1(xn)
  Delay4/1 -> AddSub3/2
  AddSub3/1 -> Delay5/1
  C7/1(yn) -> AddSub3/1
  C8/1(yn) -> Delay4/1
  C9/1(yn) -> AddSub5/1
  Delay6/1 -> AddSub5/2
  AddSub5/1 -> Delay7/1
  C10/1(yn) -> Delay6/1
  C8/2(xnz) -> [xnz]
  Delay14/1 -> AddSub13/2
  AddSub/1 -> AddSub13/1
  Delay2/1 -> AddSub2/1
  Delay5/1 -> AddSub2/2
  AddSub13/1 -> AddSub4/1
  AddSub2/1 -> AddSub4/2
  AddSub4/1 -> AddSub8/1
  C11/1(yn) -> AddSub7/1
  Delay8/1 -> AddSub7/2
  AddSub7/1 -> Delay9/1
  C12/1(yn) -> Delay8/1
  Delay7/1 -> AddSub6/1
  Delay9/1 -> AddSub6/2
  AddSub8/1 -> y/1
  C13/1(yn) -> AddSub10/1
  AddSub6/1 -> Gateway Out7/1, AddSub9/1
  AddSub9/1 -> AddSub8/2, Gateway Out8/1
